simulink model "r2_ratty2_32k_r203"  (R2012b)
settings: Solver=ode45  StartTime=0.0  StopTime=10.0  RelTol=1e-3
undefined symbols (not defined in the file): Down, pfb_coeff_gen_calc, Up
libraries: xbsIndex_r4 x20024, casper_library_misc x89, casper_library_delays x41, xps_library x14, xbsReplacements_r4 x2, simulink x1, casper_library_scopes x1
(part 127 of 207)

== system r2_ratty2_32k_r203/fft/fft_direct/butterfly1_1/twiddle/coeff_gen/cosin ==
  theta : Inport
  misci : Inport  Port=2
  Constant4 : xbsIndex_r4/Constant  const=0  gui_display_data_type=Fixed-point  arith_type=Unsigned  n_bits=1  bin_pt=0  preci_type=Single  exp_width=8  frac_width=24  explicit_period=on  period=1  equ=P=C  opselect=C  inp2=PCIN>>17  opr=+  inp1=P  carry=CIN  dbl_ovrd=off  block_type=constant
  Constant5 : xbsIndex_r4/Constant  const=0  gui_display_data_type=Fixed-point  arith_type=Unsigned  n_bits=1  bin_pt=0  preci_type=Single  exp_width=8  frac_width=24  explicit_period=on  period=1  equ=P=C  opselect=C  inp2=PCIN>>17  opr=+  inp1=P  carry=CIN  dbl_ovrd=off  block_type=constant
  Delay : xbsIndex_r4/Delay  rst=off  infoeditControl=Selection of Reset will increase slice count due to use of real FFs and instead of SRLs  en=off  latency=bram_latency  dbl_ovrd=off  reg_retiming=on  block_type=delay
  Delay10 : xbsIndex_r4/Delay  rst=off  infoeditControl=Selection of Reset will increase slice count due to use of real FFs and instead of SRLs  en=off  latency=bram_latency  dbl_ovrd=off  reg_retiming=on  block_type=delay
  Delay8 : xbsIndex_r4/Delay  rst=off  infoeditControl=Selection of Reset will increase slice count due to use of real FFs and instead of SRLs  en=off  latency=bram_latency  dbl_ovrd=off  reg_retiming=on  block_type=delay
  ROM : xbsIndex_r4/ROM  depth=2^(13)  initVector=[131072 68713054208 68706500608 68699947008 68693131264 68686577664 68680024064 68673470464 68666916864 68660363264 68653809664 68647256064 68640440320 68633886720 68627333120 68620779521 68614225921 68607672321 68601118721 68594302977 68587749377 68581195777 68574642177 68568088577 68561534977 68554981378 68548427778 68541612034 68535058434 68528504834 68521951234 68515397634 68508844034 68502290435 68495474691 68488921091 68482367491 68475813891 68469260291 68462706692 68456153092 68449599492 68442783748 68436230148 68429676549 68423122949 68416569349 68410015749 68403462150 68396908550 68390092806 68383539206 68376985607 68370432007 68363878407 68357324807 68350771208 68343955464 68337401864 68330848264 68324294665 68317741065 68311187465 68304633866 68298080266 68291264522 68284710922 68278157323 68271603723 68265050123 68258496524 68251942924 68245389324 68238573581 68232019981 68225466382 68218912782 68212359182 68205805583 68199251983 68192436239 68185882640 68179329040 68172775441 68166221841 68159668241 68153114642 68146561042 68139745299 68133191699 68126638100 68120084500 68113530900 68106977301 68100423701 68093870102 68087054358 68080500759 68073947159 68067393560 68060839960 68054286361 68047732761 68040917018 68034363418 68027809819 68021256219 68014702620 68008149020 68001595421 67995041821 67988226078 67981672478 67975118879 67968565279 67962011680 67955458080 67948904481 67942350882 67935535138 67928981539 67922427939 67915874340 67909320740 67902767141 67896213542 67889659942 67882844199 67876290599 67869737000 67863183401 67856629801 67850076202 67843522603 67836969003 67830153260 67823599661 67817046061 67810492462 67803938863 67797385263 67790831664 67784278065 67777462321 67770908722 67764355123 67757801523 67751247924 67744694325 67738140725 67731587126 67724771383 67718217784 67711664184 67705110585 67698556986 67692003387 67685449787 67678896188 67672342589 67665526846 67658973246 67652419647 67645866048 67639312449 67632758850 67626205250 67619651651 67612835908 67606282309 67599728710 67593175110 67586621511 67580067912 67573514313 67566960714 67560144971 67553591371 67547037772 67540484173 67533930574 67527376975 67520823376 67514269777 67507716178 67500900434 67494346835 67487793236 67481239637 67474686038 67468132439 67461578840 67455025241 67448209498 67441655899 67435102300 67428548701 67421995102 67415441502 67408887903 67402334304 67395780705 67388964962 67382411363 67375857764 67369304165 67362750566 67356196967 67349643368 67343089769 67336536170 67329720427 67323166828 67316613229 67310059630 67303506031 67296952432 67290398833 67283845234 67277291636 67270475893 67263922294 67257368695 67250815096 67244261497 67237707898 67231154299 67224600700 67218047101 67211231358 67204677759 67198124161 67191570562 67185016963 67178463364 67171909765 67165356166 67158802567 67151986824 67145433226 67138879627 67132326028 67125772429 67119218830 67112665231 67106111633 67099558034 67093004435 67086188692 67079635093 67073081495 67066527896 67059974297 67053420698 67046867099 67040313501 67033759902 67026944159 67020390560 67013836962 67007283363 67000729764 66994176165 66987622567 66981068968 66974515369 66967961770 66961146028 66954592429 66948038830 66941485232 66934931633 66928378034 66921824436 66915270837 66908717238 66902163640 66895610041 66888794298 66882240700 66875687101 66869133502 66862579904 66856026305 66849472706 66842919108 66836365509 66829811910 66822996168 66816442569 66809888971 66803335372 66796781773 66790228175 66783674576 66777120978 66770567379 66764013780 66757460182 66750644439 66744090841 66737537242 66730983644 66724430045 66717876447 66711322848 66704769250 66698215651 66691662053 66685108454 66678292711 66671739113 66665185514 66658631916 66652078318 66645524719 66638971121 66632417522 66625863924 66619310325 66612756727 66605940984 66599387386 66592833787 66586280189 66579726590 66573172992 66566619394 66560065795 66553512197 66546958598 66540405000 66533851402 ...(+94308 chars)]  distributed_mem=Block RAM  rst=off  init_reg=0  en=off  latency=bram_latency  gui_display_data_type=Fixed-point  arith_type=Unsigned  n_bits=n_bits*2  bin_pt=0  preci_type=Single  exp_width=8  frac_width=24  dbl_ovrd=off  optimize=Speed  use_rpm=on  block_type=sprom
  Terminator : Terminator
  Terminator1 : Terminator
  Terminator4 : Terminator
  add_convert0 : Subsystem
  add_convert1 : Subsystem
  assert : xbsIndex_r4/Assert  assert_type=on  type_source=Explicitly  gui_display_data_type=Fixed-point  arith_type=Unsigned  n_bits=13  bin_pt=0  preci_type=Single  exp_width=8  frac_width=24  assert_rate=off  rate_source=Explicitly  period=1  output_port=on  block_type=assert
  c_to_ri : casper_library_misc/c_to_ri  n_bits=n_bits  bin_pt=bin_pt
  invert0 : Subsystem  mask{n_bits=n_bits, bin_pt=bin_pt, neg_latency=neg_latency, mux_latency=mux_latency}
      mask prompts: n_bits "bit width"; bin_pt "binary point"; neg_latency "negate latency"; mux_latency "mux latency"
  invert1 : Subsystem  mask{n_bits=n_bits, bin_pt=bin_pt, neg_latency=neg_latency, mux_latency=mux_latency}
      mask prompts: n_bits "bit width"; bin_pt "binary point"; neg_latency "negate latency"; mux_latency "mux latency"
  -sin : Outport
  -cos : Outport  Port=2
  misco : Outport  Port=3
  wires:
  theta/1 -> assert/1
  assert/1 -> add_convert0/1(theta), add_convert1/1(theta)
  Constant4/1 -> add_convert0/2(misci)
  add_convert0/3(misco) -> Terminator/1
  misci/1 -> add_convert1/2(misci)
  add_convert1/3(misco) -> Delay/1
  add_convert0/2(add) -> ROM/1
  add_convert1/2(add) -> Terminator4/1
  ROM/1 -> c_to_ri/1
  add_convert1/1(negate) -> Delay8/1
  add_convert0/1(negate) -> Delay10/1
  Delay10/1 -> invert0/1(negate)
  Constant5/1 -> invert0/3(misci)
  c_to_ri/1 -> invert0/2(in)
  Delay8/1 -> invert1/1(negate)
  c_to_ri/2 -> invert1/2(in)
  invert0/2(misco) -> Terminator1/1
  Delay/1 -> invert1/3(misci)
  invert0/1(out) -> -sin/1
  invert1/2(misco) -> misco/1
  invert1/1(out) -> -cos/1
